simulink model "Simulatore_COMAU_UBHAND_IV_manipolazione_interna"  (Simulink 7.6)
settings: Solver=ode45  StartTime=0.0  StopTime=inf  RelTol=1e-3
values from neighbouring files (symbols this model uses):
  a_f = zeros(4,5)    [KinematicData.m]
  alpha_f = zeros(4,5)    [KinematicData.m]
  d_f = zeros(4,5)    [KinematicData.m]
  dc4_1 = 660    [KinematicData.m]
  T6HAND = [1 0 0 0;0 1 0 0; 0 0 1 357.48+95; 0 0 0 1]    [KinematicData.m]
  teta_d = atan(647.07/130)    [KinematicData.m]
  teta_f = zeros(4,5)    [KinematicData.m]
undefined symbols (not defined in the file or in its folder): a_c, a_w, alpha_c, alpha_w, d_c, d_w, Dt_fingers, teta_c
libraries: simulink x281, dspmtrx3 x171, aerolibutil x163, aerolibconvert2 x64, simulink_extras x46, vrlib x26, aerolibtransform2 x7, dspnetwork x1
(part 8 of 15)

== system Simulatore_COMAU_UBHAND_IV_manipolazione_interna/SCOPES/Direct_kinematic2/Direct_kinematic_wrist/T_0_8/Subsystem ==
  q : Inport
  alpha : Inport  Port=2
  a : Inport  Port=3
  A_01 : aerolibutil/Create 3x3 Matrix
  Constant : Constant  Value=0
  Constant1 : Constant  Value=[0 0 0 1]  VectorParams1D=off
  Constant5 : Constant  Value=d_w(1)
  Display : Display
  Gain : Gain  Gain=-1
  Gain1 : Gain  Gain=-1
  Matrix Concatenation : Concatenate  Mode=Multidimensional array  ConcatenateDimension=2
  Matrix Concatenation1 : Concatenate  Mode=Multidimensional array
  Product{,1..5} (6 alike) : Product  RndMeth=Zero
  Trigonometric Function{,1,6..8,11..12} (7 alike) : Trigonometry  Operator=cos
  Trigonometric Function{2..5,9..10,13} (7 alike) : Trigonometry
  Vector Concatenate : Concatenate  NumInputs=3  Mode=Multidimensional array
  Out1 : Outport
  wires:
  Matrix Concatenation1/1 -> Display/1, Out1/1
  Constant1/1 -> Matrix Concatenation1/2
  Matrix Concatenation/1 -> Matrix Concatenation1/1
  Vector Concatenate/1 -> Matrix Concatenation/2
  A_01/1 -> Matrix Concatenation/1
  Constant5/1 -> Vector Concatenate/3
  Product5/1 -> Vector Concatenate/2
  Product4/1 -> Vector Concatenate/1
  Trigonometric Function13/1 -> Product5/2
  Trigonometric Function12/1 -> Product4/2
  a/1 -> Product5/1, Product4/1
  Trigonometric Function11/1 -> A_01/9
  Trigonometric Function10/1 -> A_01/8
  Constant/1 -> A_01/7
  Gain1/1 -> A_01/6
  Product2/1 -> A_01/5
  Trigonometric Function5/1 -> A_01/4
  Product1/1 -> A_01/3
  Gain/1 -> A_01/2
  Trigonometric Function/1 -> A_01/1
  Product/1 -> Gain/1
  Product3/1 -> Gain1/1
  Trigonometric Function9/1 -> Product3/2
  Trigonometric Function8/1 -> Product3/1
  Trigonometric Function7/1 -> Product2/2
  Trigonometric Function6/1 -> Product2/1
  Trigonometric Function4/1 -> Product1/2
  Trigonometric Function3/1 -> Product1/1
  alpha/1 -> Trigonometric Function11/1, Trigonometric Function10/1, Trigonometric Function9/1, Trigonometric Function7/1, Trigonometric Function4/1, Trigonometric Function1/1
  q/1 -> Trigonometric Function13/1, Trigonometric Function12/1, Trigonometric Function8/1, Trigonometric Function6/1, Trigonometric Function5/1, Trigonometric Function3/1, Trigonometric Function2/1, Trigonometric Function/1
  Trigonometric Function1/1 -> Product/2
  Trigonometric Function2/1 -> Product/1

== system Simulatore_COMAU_UBHAND_IV_manipolazione_interna/SCOPES/Direct_kinematic2/Direct_kinematic_wrist/T_0_8/Subsystem1 ==
  q : Inport
  alpha : Inport  Port=2
  a : Inport  Port=3
  A_01 : aerolibutil/Create 3x3 Matrix
  Constant : Constant  Value=0
  Constant1 : Constant  Value=[0 0 0 1]  VectorParams1D=off
  Constant5 : Constant  Value=d_w(2)
  Display : Display
  Gain : Gain  Gain=-1
  Gain1 : Gain  Gain=-1
  Matrix Concatenation : Concatenate  Mode=Multidimensional array  ConcatenateDimension=2
  Matrix Concatenation1 : Concatenate  Mode=Multidimensional array
  Product{,1..5} (6 alike) : Product  RndMeth=Zero
  Trigonometric Function{,1,6..8,11..12} (7 alike) : Trigonometry  Operator=cos
  Trigonometric Function{2..5,9..10,13} (7 alike) : Trigonometry
  Vector Concatenate : Concatenate  NumInputs=3  Mode=Multidimensional array
  Out1 : Outport
  wires:
  Trigonometric Function2/1 -> Product/1
  Trigonometric Function1/1 -> Product/2
  q/1 -> Trigonometric Function/1, Trigonometric Function2/1, Trigonometric Function3/1, Trigonometric Function5/1, Trigonometric Function6/1, Trigonometric Function8/1, Trigonometric Function12/1, Trigonometric Function13/1
  alpha/1 -> Trigonometric Function1/1, Trigonometric Function4/1, Trigonometric Function7/1, Trigonometric Function9/1, Trigonometric Function10/1, Trigonometric Function11/1
  Trigonometric Function3/1 -> Product1/1
  Trigonometric Function4/1 -> Product1/2
  Trigonometric Function6/1 -> Product2/1
  Trigonometric Function7/1 -> Product2/2
  Trigonometric Function8/1 -> Product3/1
  Trigonometric Function9/1 -> Product3/2
  Product3/1 -> Gain1/1
  Product/1 -> Gain/1
  Trigonometric Function/1 -> A_01/1
  Gain/1 -> A_01/2
  Product1/1 -> A_01/3
  Trigonometric Function5/1 -> A_01/4
  Product2/1 -> A_01/5
  Gain1/1 -> A_01/6
  Constant/1 -> A_01/7
  Trigonometric Function10/1 -> A_01/8
  Trigonometric Function11/1 -> A_01/9
  a/1 -> Product5/1, Product4/1
  Trigonometric Function12/1 -> Product4/2
  Trigonometric Function13/1 -> Product5/2
  Product4/1 -> Vector Concatenate/1
  Product5/1 -> Vector Concatenate/2
  Constant5/1 -> Vector Concatenate/3
  A_01/1 -> Matrix Concatenation/1
  Vector Concatenate/1 -> Matrix Concatenation/2
  Matrix Concatenation/1 -> Matrix Concatenation1/1
  Constant1/1 -> Matrix Concatenation1/2
  Matrix Concatenation1/1 -> Out1/1, Display/1

== system Simulatore_COMAU_UBHAND_IV_manipolazione_interna/SCOPES/Direct_kinematic2/Direct_kinematic_wrist/T_0_8/wrist_reference_ ==
  Angle Conversion : aerolibconvert2/Angle Conversion  IU=deg  OU=rad
  Constant1 : Constant  Value=[0 90 0]  VectorParams1D=off
  Constant2 : Constant  Value=[0 0 0 ]'  VectorParams1D=off
  Constant4 : Constant  Value=[0 0 0 1]  VectorParams1D=off
  Matrix Concatenate : Concatenate  Mode=Multidimensional array  ConcatenateDimension=2
  Matrix Concatenate1 : Concatenate  Mode=Multidimensional array
  Rotation Angles to Direction Cosine Matrix : aerolibtransform2/Rotation Angles to Direction Cosine Matrix  rotationOrder=ZYX
  T0_W : Outport
  wires:
  Matrix Concatenate1/1 -> T0_W/1
  Rotation Angles to Direction Cosine Matrix/1 -> Matrix Concatenate/1
  Constant4/1 -> Matrix Concatenate1/2
  Matrix Concatenate/1 -> Matrix Concatenate1/1
  Angle Conversion/1 -> Rotation Angles to Direction Cosine Matrix/1
  Constant1/1 -> Angle Conversion/1
  Constant2/1 -> Matrix Concatenate/2

== system Simulatore_COMAU_UBHAND_IV_manipolazione_interna/Work Space Control/Grasp Planner/SCOPES/Direct_kinematic2/Direct_kinematic_Comau ==
  q[1..6] : Inport
  Demux3 : Demux  Outputs=6
  Matrix Multiply : Product  Multiplication=Matrix(*)
  Mux : Mux  Inputs=3
  Mux2 : Mux  Inputs=3
  T_0_3 : Subsystem
  T_3_6 : Subsystem
  T_0_6 : Outport
  wires:
  Demux3/1 -> Mux/1
  Demux3/2 -> Mux/2
  Demux3/3 -> Mux/3
  Mux/1 -> T_0_3/1(q[1..3])
  Demux3/4 -> Mux2/1
  Demux3/5 -> Mux2/2
  Demux3/6 -> Mux2/3
  Mux2/1 -> T_3_6/1(q[1..3])
  T_3_6/1(T_3_6) -> Matrix Multiply/2
  q[1..6]/1 -> Demux3/1
  T_0_3/1(T_0_3) -> Matrix Multiply/1
  Matrix Multiply/1 -> T_0_6/1

== system Simulatore_COMAU_UBHAND_IV_manipolazione_interna/Work Space Control/Grasp Planner/SCOPES/Direct_kinematic2/Direct_kinematic_hand ==
  Joint_references : Inport
  T_08 : Inport  Port=2
  Constant : Constant  Value=Dt_fingers(1,1).T_w2_finger_base  VectorParams1D=off
  Constant1 : Constant  Value=Dt_fingers(1,2).T_w2_finger_base  VectorParams1D=off
  Constant2 : Constant  Value=Dt_fingers(1,3).T_w2_finger_base  VectorParams1D=off
  Constant3 : Constant  Value=Dt_fingers(1,4).T_w2_finger_base  VectorParams1D=off
  Constant4 : Constant  Value=Dt_fingers(1,5).T_w2_finger_base  VectorParams1D=off
  Demux : Demux  Outputs=5
  Direct_kinematic_index : Subsystem
  Direct_kinematic_little : Subsystem
  Direct_kinematic_middle : Subsystem
  Direct_kinematic_ring : Subsystem
  Direct_kinematic_thumb : Subsystem
  Matrix Multiply{,1..4} (5 alike) : Product  Multiplication=Matrix(*)
  xyz{,1..4} (5 alike) : dspmtrx3/Submatrix  RowSpan=Range of rows  RowStartMode=Index  RowStartIndex=1  RowEndMode=Index  RowEndIndex=4  ColSpan=One column  ColStartMode=Index  ColStartIndex=4  ColEndMode=Index  ColEndIndex=3
  T_finger_tip_thumb : Outport
  T_finger_tip_index : Outport  Port=2
  T_finger_tip_middle : Outport  Port=3
  T_finger_tip_ring : Outport  Port=4
  T_finger_tip_little : Outport  Port=5
  T_thumb_base : Outport  Port=6
  wires:
  T_08/1 -> Matrix Multiply/1, Matrix Multiply1/1, Matrix Multiply2/1, Matrix Multiply3/1, Matrix Multiply4/1
  Constant/1 -> Matrix Multiply/2
  Constant1/1 -> Matrix Multiply1/2
  Constant2/1 -> Matrix Multiply2/2
  Constant3/1 -> Matrix Multiply3/2
  Constant4/1 -> Matrix Multiply4/2
  Matrix Multiply/1 -> Direct_kinematic_thumb/2(T_0f)
  Matrix Multiply1/1 -> Direct_kinematic_index/2(T_0f)
  Matrix Multiply2/1 -> Direct_kinematic_middle/2(T_0f)
  Matrix Multiply3/1 -> Direct_kinematic_ring/2(T_0f)
  Matrix Multiply4/1 -> Direct_kinematic_little/2(T_0f)
  Joint_references/1 -> Demux/1
  Demux/1 -> Direct_kinematic_thumb/1(Joints_reference)
  Demux/2 -> Direct_kinematic_index/1(Joints_reference)
  Demux/3 -> Direct_kinematic_middle/1(Joints_reference)
  Demux/4 -> Direct_kinematic_ring/1(Joints_reference)
  Demux/5 -> Direct_kinematic_little/1(Joints_reference)
  Direct_kinematic_index/1(T_finger_tip) -> xyz1/1
  Direct_kinematic_middle/1(T_finger_tip) -> xyz2/1
  Direct_kinematic_ring/1(T_finger_tip) -> xyz3/1
  Direct_kinematic_little/1(T_finger_tip) -> xyz4/1
  Direct_kinematic_thumb/1(T_finger_tip) -> xyz/1
  Direct_kinematic_thumb/2(T_thumb_base) -> T_thumb_base/1
  xyz1/1 -> T_finger_tip_index/1
  xyz/1 -> T_finger_tip_thumb/1
  xyz2/1 -> T_finger_tip_middle/1
  xyz3/1 -> T_finger_tip_ring/1
  xyz4/1 -> T_finger_tip_little/1

== system Simulatore_COMAU_UBHAND_IV_manipolazione_interna/Work Space Control/Grasp Planner/SCOPES/Direct_kinematic2/Direct_kinematic_wrist ==
  wrist_angles[1..2] : Inport
  T_0HAND : Inport  Port=2
  Demux3 : Demux  Outputs=2
  Mux : Mux  Inputs=2
  T_0_8 : Subsystem
  T_08 : Outport
  wires:
  T_0_8/1(T_08) -> T_08/1
  wrist_angles[1..2]/1 -> Demux3/1
  Mux/1 -> T_0_8/1(wrist_angles[1..2])
  Demux3/2 -> Mux/2
  Demux3/1 -> Mux/1
  T_0HAND/1 -> T_0_8/2(T_0HAND)

== system Simulatore_COMAU_UBHAND_IV_manipolazione_interna/Work Space Control/Grasp Planner/box/presa da destra/wrist index angles des2 ==
  Constant : Constant
  Index_medial_des : Constant  Value=80
  Index_proximal_back_forward_des : Constant  Value=50
  Index_proximal_left_right_des : Constant  Value=0
  Mux1 : Mux  Inputs=2
  Mux2 : Mux  Inputs=3
  wrist_back_forward_des : simulink/Math Operations/Slider Gain  low=-180  gain=0  high=180
  wrist_left_right_des : simulink/Math Operations/Slider Gain  low=-180  gain=0  high=180
  wrist_des : Outport
  index_des : Outport  Port=2
  wires:
  [one] -> wrist_left_right_des/1
  wrist_left_right_des/1 -> Mux1/1
  [one] -> wrist_back_forward_des/1
  wrist_back_forward_des/1 -> Mux1/2
  Index_proximal_left_right_des/1 -> Mux2/1
  Index_medial_des/1 -> Mux2/3
  Index_proximal_back_forward_des/1 -> Mux2/2
  Mux2/1 -> index_des/1
  Mux1/1 -> wrist_des/1
  Constant/1 -> [one]

== system Simulatore_COMAU_UBHAND_IV_manipolazione_interna/Work Space Control/Grasp Planner/box/presa dal basso/wrist index angles des2 ==
  Constant : Constant
  Index_medial_des : Constant  Value=70
  Index_proximal_back_forward_des : Constant  Value=70
  Index_proximal_left_right_des : Constant  Value=0
  Mux1 : Mux  Inputs=2
  Mux2 : Mux  Inputs=3
  wrist_back_forward_des : simulink/Math Operations/Slider Gain  low=-180  gain=0  high=180
  wrist_left_right_des : simulink/Math Operations/Slider Gain  low=-180  gain=0  high=180
  wrist_des : Outport
  index_des : Outport  Port=2
  wires:
  [one] -> wrist_left_right_des/1
  wrist_left_right_des/1 -> Mux1/1
  [one] -> wrist_back_forward_des/1
  wrist_back_forward_des/1 -> Mux1/2
  Index_proximal_left_right_des/1 -> Mux2/1
  Index_medial_des/1 -> Mux2/3
  Index_proximal_back_forward_des/1 -> Mux2/2
  Mux2/1 -> index_des/1
  Mux1/1 -> wrist_des/1
  Constant/1 -> [one]

== system Simulatore_COMAU_UBHAND_IV_manipolazione_interna/Work Space Control/Grasp Planner/box/presa dall'alto/wrist index angles des2 ==
  Constant : Constant
  Index_medial_des : Constant  Value=20
  Index_proximal_back_forward_des : Constant  Value=40
  Index_proximal_left_right_des : Constant  Value=5
  Mux1 : Mux  Inputs=2
  Mux2 : Mux  Inputs=3
  wrist_back_forward_des : simulink/Math Operations/Slider Gain  low=-180  gain=15  high=180
  wrist_left_right_des : simulink/Math Operations/Slider Gain  low=-180  gain=0  high=180
  wrist_des : Outport
  index_des : Outport  Port=2
  wires:
  [one] -> wrist_left_right_des/1
  wrist_left_right_des/1 -> Mux1/1
  [one] -> wrist_back_forward_des/1
  wrist_back_forward_des/1 -> Mux1/2
  Index_proximal_left_right_des/1 -> Mux2/1
  Index_medial_des/1 -> Mux2/3
  Index_proximal_back_forward_des/1 -> Mux2/2
  Mux2/1 -> index_des/1
  Mux1/1 -> wrist_des/1
  Constant/1 -> [one]

== system Simulatore_COMAU_UBHAND_IV_manipolazione_interna/Work Space Control/Grasp Planner/box/punti di contatto box/object rotation matrix ==
  box_pos : Inport
  object_hand_relative_orientation : Inport  Port=2
  object_motion_parameters : Inport  Port=3
  Constant : Constant
  Constant1 : Constant  Value=[0 0 0 1]  VectorParams1D=off
  Constant2 : Constant  Value=[0 0 0 1]  VectorParams1D=off
  Demux : Demux  Outputs=12
  Demux1 : Demux  Outputs=3
  Display{,1..3} (4 alike) : Display
  Gain : Gain  Gain=-1
  Matrix Concatenate : Concatenate  Mode=Multidimensional array  ConcatenateDimension=2
  Matrix Concatenate1 : Concatenate  Mode=Multidimensional array
  Matrix Concatenate2 : Concatenate  Mode=Multidimensional array  ConcatenateDimension=2
  Matrix Concatenate3 : Concatenate  Mode=Multidimensional array
  Matrix Multiply3 : Product  Multiplication=Matrix(*)
  Multiport Switch1 : MultiPortSwitch  Inputs=2  zeroidx=off
  Mux : Mux  Inputs=3
  Mux1 : Mux  Inputs=3
  R : Subsystem
  R_01 : Subsystem
  R_56 : Subsystem
  Ramp : simulink/Sources/Ramp  slope=50  start=0  X0=0
  definizione_T_0_object_tip1 : Subsystem
  T_object_center : Outport
  tag scope: R_0_end_effector_box=global, R_box=global, T_0_object_tip_box=global, T_rot_center_box=global, box_pos=global
  wires:
  Constant/1 -> [one]
  Constant1/1 -> Matrix Concatenate1/2
  Matrix Concatenate/1 -> Matrix Concatenate1/1
  box_pos/1 -> definizione_T_0_object_tip1/1(box_pos)
  Demux1/1 -> R_56/1(alpha)
  Demux1/2 -> R_56/2(beta)
  Demux1/3 -> R_56/3(gamma)
  object_hand_relative_orientation/1 -> Demux1/1
  object_motion_parameters/1 -> Demux/1
  Demux/1 -> R_01/1(alpha)
  Demux/2 -> R_01/2(beta)
  Demux/3 -> R_01/3(gamma)
  Demux/4 -> Mux/1
  Demux/5 -> Mux/2
  Demux/6 -> Mux/3
  Demux/7 -> Mux1/1
  Demux/8 -> Mux1/2
  Demux/9 -> Mux1/3
  R_01/1(R_01) -> definizione_T_0_object_tip1/2(R_01), [R_01]
  Mux/1 -> definizione_T_0_object_tip1/3(1pc)
  Mux1/1 -> definizione_T_0_object_tip1/4(2pr), Gain/1
  Gain/1 -> definizione_T_0_object_tip1/5(3pc)
  R_56/1(R56) -> Matrix Multiply3/2
  Matrix Multiply3/1 -> [R_0_end_effector_box]
  definizione_T_0_object_tip1/1(0pc) -> Display3/1
  definizione_T_0_object_tip1/3(0pc') -> [box_pos], Matrix Concatenate2/2
  Matrix Concatenate1/1 -> [T_rot_center_box], Display/1
  definizione_T_0_object_tip1/2(0pr) -> Matrix Concatenate/2, Display1/1
  [orientation_mode_box] -> Multiport Switch1/1
  Multiport Switch1/1 -> [R_box], Matrix Concatenate2/1
  [R_01] -> Multiport Switch1/2
  [R_05] -> Multiport Switch1/3
  Constant2/1 -> Matrix Concatenate3/2
  Matrix Concatenate2/1 -> Matrix Concatenate3/1
  Matrix Concatenate3/1 -> [T_0_object_tip_box], T_object_center/1, Display2/1
  [R_box] -> Matrix Multiply3/1
  R/1(R) -> definizione_T_0_object_tip1/6(R)
  definizione_T_0_object_tip1/5(R_05) -> [R_05]
  Demux/10 -> R/1(alpha)
  Demux/11 -> R/2(beta)
  Demux/12 -> R/3(gamma)
  definizione_T_0_object_tip1/4(R1) -> Matrix Concatenate/1

== system Simulatore_COMAU_UBHAND_IV_manipolazione_interna/Work Space Control/Grasp Planner/box/punti di contatto box/punti di contatto sfera ==
  teta/z thumb : Inport
  teta/z index : Inport  Port=2
  teta/z middle : Inport  Port=3
  teta/z ring : Inport  Port=4
  teta/z little : Inport  Port=5
  box_size : Inport  Port=6
  T_object : Inport  Port=7
  Mux : Mux  Inputs=5
  Subsystem : Subsystem
  Subsystem1 : Subsystem
  Subsystem2 : Subsystem
  Subsystem3 : Subsystem
  Subsystem4 : Subsystem
  contact_tips_xyz : Outport
  tag scope: box_size=global
  wires:
  teta/z thumb/1 -> Subsystem/1(dimens1/2/side)
  box_size/1 -> [box_size]
  teta/z middle/1 -> Subsystem2/1(dimens1/2/side)
  teta/z ring/1 -> Subsystem3/1(dimens1/2/side)
  teta/z little/1 -> Subsystem4/1(dimens1/2/side)
  Subsystem/1(xyz 0) -> Mux/1
  Subsystem1/1(xyz 0) -> Mux/2
  Subsystem2/1(xyz 0) -> Mux/3
  Subsystem3/1(xyz 0) -> Mux/4
  Subsystem4/1(xyz 0) -> Mux/5
  Mux/1 -> contact_tips_xyz/1
  T_object/1 -> Subsystem3/2(T_object), Subsystem4/2(T_object), Subsystem2/2(T_object), Subsystem1/2(T_object), Subsystem/2(T_object)
  teta/z index/1 -> Subsystem1/1(dimens1/2/side)

== system Simulatore_COMAU_UBHAND_IV_manipolazione_interna/Work Space Control/Grasp Planner/cilindro/Subsystem/R_end_effector ==
  alpha,beta,gamma : Inport
  Demux : Demux  Outputs=3
  Matrix Multiply3 : Product  Multiplication=Matrix(*)
  Matrix Multiply4 : Product  Multiplication=Matrix(*)
  rot_x : Subsystem
  rot_y : Subsystem
  rot_z : Subsystem
  R_end_effector : Outport
  wires:
  Matrix Multiply4/1 -> R_end_effector/1
  Matrix Multiply3/1 -> Matrix Multiply4/1
  rot_z/1(rotz) -> Matrix Multiply4/2
  rot_y/1(roty) -> Matrix Multiply3/2
  rot_x/1(rotx) -> Matrix Multiply3/1
  Demux/1 -> rot_x/1(alpha)
  Demux/2 -> rot_y/1(beta)
  Demux/3 -> rot_z/1(gamma)
  alpha,beta,gamma/1 -> Demux/1

== system Simulatore_COMAU_UBHAND_IV_manipolazione_interna/Work Space Control/Grasp Planner/cilindro/presa da destra/wrist index angles des2 ==
  Constant : Constant
  Index_medial_des : Constant  Value=50
  Index_proximal_back_forward_des : Constant  Value=30
  Index_proximal_left_right_des : Constant  Value=10
  Mux1 : Mux  Inputs=2
  Mux2 : Mux  Inputs=3
  wrist_back_forward_des : simulink/Math Operations/Slider Gain  low=-180  gain=0  high=180
  wrist_left_right_des : simulink/Math Operations/Slider Gain  low=-180  gain=0  high=180
  wrist_des : Outport
  index_des : Outport  Port=2
  wires:
  [one] -> wrist_left_right_des/1
  wrist_left_right_des/1 -> Mux1/1
  [one] -> wrist_back_forward_des/1
  wrist_back_forward_des/1 -> Mux1/2
  Index_proximal_left_right_des/1 -> Mux2/1
  Index_medial_des/1 -> Mux2/3
  Index_proximal_back_forward_des/1 -> Mux2/2
  Mux2/1 -> index_des/1
  Mux1/1 -> wrist_des/1
  Constant/1 -> [one]

== system Simulatore_COMAU_UBHAND_IV_manipolazione_interna/Work Space Control/Grasp Planner/cilindro/presa dal basso/wrist index angles des2 ==
  Constant : Constant
  Index_medial_des : Constant  Value=15
  Index_proximal_back_forward_des : Constant  Value=80
  Index_proximal_left_right_des : Constant  Value=0
  Mux1 : Mux  Inputs=2
  Mux2 : Mux  Inputs=3
  wrist_back_forward_des : simulink/Math Operations/Slider Gain  low=-180  gain=0  high=180
  wrist_left_right_des : simulink/Math Operations/Slider Gain  low=-180  gain=0  high=180
  wrist_des : Outport
  index_des : Outport  Port=2
  wires:
  [one] -> wrist_left_right_des/1
  wrist_left_right_des/1 -> Mux1/1
  [one] -> wrist_back_forward_des/1
  wrist_back_forward_des/1 -> Mux1/2
  Index_proximal_left_right_des/1 -> Mux2/1
  Index_medial_des/1 -> Mux2/3
  Index_proximal_back_forward_des/1 -> Mux2/2
  Mux2/1 -> index_des/1
  Mux1/1 -> wrist_des/1
  Constant/1 -> [one]

== system Simulatore_COMAU_UBHAND_IV_manipolazione_interna/Work Space Control/Grasp Planner/cilindro/presa dall'alto/wrist index angles des2 ==
  Constant : Constant
  Index_medial_des : Constant  Value=50
  Index_proximal_back_forward_des : Constant  Value=20
  Index_proximal_left_right_des : Constant  Value=10
  Mux1 : Mux  Inputs=2
  Mux2 : Mux  Inputs=3
  wrist_back_forward_des : simulink/Math Operations/Slider Gain  low=-180  gain=28.8  high=180
  wrist_left_right_des : simulink/Math Operations/Slider Gain  low=-180  gain=0  high=180
  wrist_des : Outport
  index_des : Outport  Port=2
  wires:
  [one] -> wrist_left_right_des/1
  wrist_left_right_des/1 -> Mux1/1
  [one] -> wrist_back_forward_des/1
  wrist_back_forward_des/1 -> Mux1/2
  Index_proximal_left_right_des/1 -> Mux2/1
  Index_medial_des/1 -> Mux2/3
  Index_proximal_back_forward_des/1 -> Mux2/2
  Mux2/1 -> index_des/1
  Mux1/1 -> wrist_des/1
  Constant/1 -> [one]

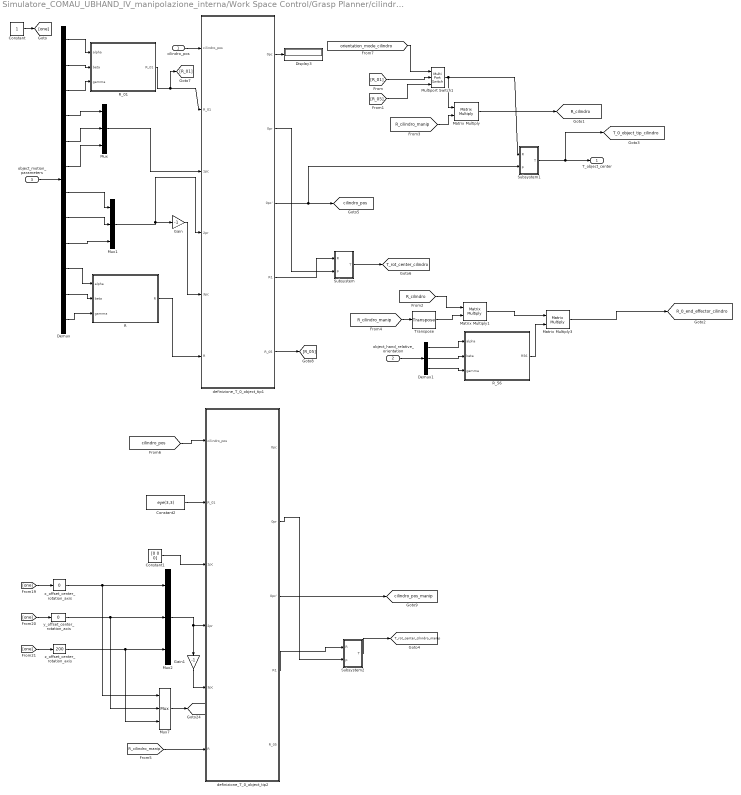
== system Simulatore_COMAU_UBHAND_IV_manipolazione_interna/Work Space Control/Grasp Planner/cilindro/punti di contatto cilindro/object rotation matrix ==
  cilindro_pos : Inport
  object_hand_relative_orientation : Inport  Port=2
  object_motion_parameters : Inport  Port=3
  Constant : Constant
  Constant1 : Constant  Value=[0 0 0]
  Constant2 : Constant  Value=eye(3,3)
  Demux : Demux  Outputs=12
  Demux1 : Demux  Outputs=3
  Display3 : Display
  Gain : Gain  Gain=-1
  Gain1 : Gain  Gain=-1
  Matrix Multiply : Product  Multiplication=Matrix(*)
  Matrix Multiply1 : Product  Multiplication=Matrix(*)
  Matrix Multiply3 : Product  Multiplication=Matrix(*)
  Multiport Switch1 : MultiPortSwitch  Inputs=2  zeroidx=off
  Mux{,1..2,7} (4 alike) : Mux  Inputs=3
  R : Subsystem
  R_01 : Subsystem
  R_56 : Subsystem
  Subsystem : Subsystem
  Subsystem1 : Subsystem
  Subsystem2 : Subsystem
  Transpose : dspmtrx3/Transpose  Hermitian=off  overflowFlag=off
  definizione_T_0_object_tip1 : Subsystem
  definizione_T_0_object_tip2 : Subsystem
  x_offset_center_rotation_axis : simulink/Math Operations/Slider Gain  low=0  gain=0  high=500
  y_offset_center_rotation_axis : simulink/Math Operations/Slider Gain  low=0  gain=0  high=500
  z_offset_center_rotation_axis : simulink/Math Operations/Slider Gain  low=-1000  gain=200  high=500
  T_object_center : Outport
  tag scope: R_0_end_effector_cilindro=global, R_cilindro=global, T_0_object_tip_cilindro=global, T_rot_center_cilindro=global, T_rot_center_cilindro_manip=global, cilindro_pos=global, cilindro_pos_manip=global, reference_point=global
  wires:
  Constant/1 -> [one]
  cilindro_pos/1 -> definizione_T_0_object_tip1/1(cilindro_pos)
  Demux1/1 -> R_56/1(alpha)
  Demux1/2 -> R_56/2(beta)
  Demux1/3 -> R_56/3(gamma)
  object_hand_relative_orientation/1 -> Demux1/1
  object_motion_parameters/1 -> Demux/1
  Demux/4 -> Mux/1
  Demux/5 -> Mux/2
  Demux/6 -> Mux/3
  Demux/7 -> Mux1/1
  Demux/8 -> Mux1/2
  Demux/9 -> Mux1/3
  R_01/1(R_01) -> definizione_T_0_object_tip1/2(R_01), [R_01]
  Mux/1 -> definizione_T_0_object_tip1/3(1pc)
  Mux1/1 -> definizione_T_0_object_tip1/4(2pr), Gain/1
  Gain/1 -> definizione_T_0_object_tip1/5(3pc)
  R_56/1(R56) -> Matrix Multiply3/2
  Matrix Multiply3/1 -> [R_0_end_effector_cilindro]
  definizione_T_0_object_tip1/1(0pc) -> Display3/1
  definizione_T_0_object_tip1/3(0pc') -> Subsystem1/2(p), [cilindro_pos]
  Subsystem/1(T) -> [T_rot_center_cilindro]
  definizione_T_0_object_tip1/2(0pr) -> Subsystem/2(p)
  [orientation_mode_cilindro] -> Multiport Switch1/1
  [R_01] -> Multiport Switch1/2
  [R_05] -> Multiport Switch1/3
  Subsystem1/1(T) -> [T_0_object_tip_cilindro], T_object_center/1
  R/1(R) -> definizione_T_0_object_tip1/6(R)
  definizione_T_0_object_tip1/5(R_05) -> [R_05]
  Demux/10 -> R/1(alpha)
  Demux/11 -> R/2(beta)
  Demux/12 -> R/3(gamma)
  definizione_T_0_object_tip1/4(R1) -> Subsystem/1(R)
  [R_cilindro_manip] -> Matrix Multiply/2
  Multiport Switch1/1 -> Matrix Multiply/1, Subsystem1/1(R)
  Matrix Multiply/1 -> [R_cilindro]
  [R_cilindro_manip] -> Transpose/1
  Matrix Multiply1/1 -> Matrix Multiply3/1
  [R_cilindro] -> Matrix Multiply1/1
  Transpose/1 -> Matrix Multiply1/2
  Demux/1 -> R_01/1(alpha)
  Demux/2 -> R_01/2(beta)
  Demux/3 -> R_01/3(gamma)
  [one] -> x_offset_center_rotation_axis/1
  [one] -> y_offset_center_rotation_axis/1
  [one] -> z_offset_center_rotation_axis/1
  x_offset_center_rotation_axis/1 -> Mux2/1, Mux7/1
  y_offset_center_rotation_axis/1 -> Mux2/2, Mux7/2
  z_offset_center_rotation_axis/1 -> Mux2/3, Mux7/3
  Mux2/1 -> definizione_T_0_object_tip2/4(2pr), Gain1/1
  Gain1/1 -> definizione_T_0_object_tip2/5(3pc)
  [R_cilindro_manip] -> definizione_T_0_object_tip2/6(R)
  Constant2/1 -> definizione_T_0_object_tip2/2(R_01)
  definizione_T_0_object_tip2/3(0pc') -> [cilindro_pos_manip]
  Mux7/1 -> [reference_point]
  Constant1/1 -> definizione_T_0_object_tip2/3(1pc)
  [cilindro_pos] -> definizione_T_0_object_tip2/1(cilindro_pos)
  Subsystem2/1(T) -> [T_rot_center_cilindro_manip]
  definizione_T_0_object_tip2/2(0pr) -> Subsystem2/2(p)
  definizione_T_0_object_tip2/4(R1) -> Subsystem2/1(R)

== system Simulatore_COMAU_UBHAND_IV_manipolazione_interna/Work Space Control/Grasp Planner/cilindro/punti di contatto cilindro/punti di contatto cilindro ==
  teta/z thumb : Inport
  teta/z index : Inport  Port=2
  teta/z middle : Inport  Port=3
  teta/z ring : Inport  Port=4
  teta/z little : Inport  Port=5
  cilindro_size : Inport  Port=6
  T_object : Inport  Port=7
  offset : Inport  Port=8
  object_manipulation : Inport  Port=9
  Display : Display
  Display1 : Display
  Mux : Mux  Inputs=5
  Subsystem1 : Subsystem
  Subsystem2 : Subsystem
  Subsystem3 : Subsystem
  Subsystem4 : Subsystem
  Subsystem5 : Subsystem
  contact_tips_xyz : Outport
  index_xyz_0 : Outport  Port=2
  tag scope: cilindro_size=global
  wires:
  teta/z thumb/1 -> Subsystem2/1(teta/z)
  cilindro_size/1 -> [cilindro_size]
  teta/z index/1 -> Subsystem1/1(teta/z)
  Mux/1 -> contact_tips_xyz/1, Display/1
  teta/z middle/1 -> Subsystem3/1(teta/z)
  teta/z ring/1 -> Subsystem4/1(teta/z)
  teta/z little/1 -> Subsystem5/1(teta/z)
  T_object/1 -> Subsystem1/2(T_object), Subsystem2/2(T_object), Subsystem3/2(T_object), Subsystem4/2(T_object), Subsystem5/2(T_object)
  offset/1 -> Subsystem2/3(offset), Subsystem1/3(offset), Subsystem3/3(offset), Subsystem4/3(offset), Subsystem5/3(offset)
  Subsystem2/1(xyz 0) -> Mux/1
  Subsystem5/1(xyz 0) -> Mux/5
  Subsystem4/1(xyz 0) -> Mux/4
  Subsystem3/1(xyz 0) -> Mux/3
  Subsystem1/2(xyz 0) -> Mux/2
  Subsystem1/1(index_xyz_0) -> index_xyz_0/1, Display1/1
  object_manipulation/1 -> Subsystem2/4(object_manipulation), Subsystem4/4(object_manipulation), Subsystem5/4(object_manipulation), Subsystem3/4(object_manipulation), Subsystem1/4(object_manipulation)

== system Simulatore_COMAU_UBHAND_IV_manipolazione_interna/Work Space Control/Grasp Planner/sfera/presa dall'alto/wrist index angles des2 ==
  Constant : Constant
  Index_medial_des : Constant  Value=30
  Index_proximal_back_forward_des : Constant  Value=0
  Index_proximal_left_right_des : Constant  Value=20
  Mux1 : Mux  Inputs=2
  Mux2 : Mux  Inputs=3
  wrist_back_forward_des : simulink/Math Operations/Slider Gain  low=-180  gain=0  high=180
  wrist_left_right_des : simulink/Math Operations/Slider Gain  low=-180  gain=0  high=180
  wrist_des : Outport
  index_des : Outport  Port=2
  wires:
  Constant/1 -> [one]
  Mux1/1 -> wrist_des/1
  Mux2/1 -> index_des/1
  Index_proximal_back_forward_des/1 -> Mux2/2
  Index_medial_des/1 -> Mux2/3
  Index_proximal_left_right_des/1 -> Mux2/1
  wrist_back_forward_des/1 -> Mux1/2
  [one] -> wrist_back_forward_des/1
  wrist_left_right_des/1 -> Mux1/1
  [one] -> wrist_left_right_des/1

== system Simulatore_COMAU_UBHAND_IV_manipolazione_interna/Work Space Control/Grasp Planner/sfera/punti di contatto sfera/object rotation matrix ==
  sfera_pos : Inport
  object_hand_relative_orientation : Inport  Port=2
  object_motion_parameters : Inport  Port=3
  Constant : Constant
  Constant1 : Constant  Value=[0 0 0 1]  VectorParams1D=off
  Constant2 : Constant  Value=[0 0 0 1]  VectorParams1D=off
  Demux : Demux  Outputs=12
  Demux1 : Demux  Outputs=3
  Display{,1..3} (4 alike) : Display
  Gain : Gain  Gain=-1
  Matrix Concatenate : Concatenate  Mode=Multidimensional array  ConcatenateDimension=2
  Matrix Concatenate1 : Concatenate  Mode=Multidimensional array
  Matrix Concatenate2 : Concatenate  Mode=Multidimensional array  ConcatenateDimension=2
  Matrix Concatenate3 : Concatenate  Mode=Multidimensional array
  Matrix Multiply3 : Product  Multiplication=Matrix(*)
  Multiport Switch1 : MultiPortSwitch  Inputs=2  zeroidx=off
  Mux : Mux  Inputs=3
  Mux1 : Mux  Inputs=3
  R : Subsystem
  R_01 : Subsystem
  R_56 : Subsystem
  Ramp : simulink/Sources/Ramp  slope=50  start=0  X0=0
  definizione_T_0_object_tip1 : Subsystem
  T_object_center : Outport
  tag scope: R_0_end_effector_sfera=global, R_sfera=global, T_0_object_tip_sfera=global, T_rot_center_sfera=global, sfera_pos=global
  wires:
  Constant/1 -> [one]
  Constant1/1 -> Matrix Concatenate1/2
  Matrix Concatenate/1 -> Matrix Concatenate1/1
  sfera_pos/1 -> definizione_T_0_object_tip1/1(sfera_pos)
  Demux1/1 -> R_56/1(alpha)
  Demux1/2 -> R_56/2(beta)
  Demux1/3 -> R_56/3(gamma)
  object_hand_relative_orientation/1 -> Demux1/1
  object_motion_parameters/1 -> Demux/1
  Demux/1 -> R_01/1(alpha)
  Demux/2 -> R_01/2(beta)
  Demux/3 -> R_01/3(gamma)
  Demux/4 -> Mux/1
  Demux/5 -> Mux/2
  Demux/6 -> Mux/3
  Demux/7 -> Mux1/1
  Demux/8 -> Mux1/2
  Demux/9 -> Mux1/3
  R_01/1(R_01) -> definizione_T_0_object_tip1/2(R_01), [R_01]
  Mux/1 -> definizione_T_0_object_tip1/3(1pc)
  Mux1/1 -> definizione_T_0_object_tip1/4(2pr), Gain/1
  Gain/1 -> definizione_T_0_object_tip1/5(3pc)
  R_56/1(R56) -> Matrix Multiply3/2
  Matrix Multiply3/1 -> [R_0_end_effector_sfera]
  definizione_T_0_object_tip1/1(0pc) -> Display3/1
  definizione_T_0_object_tip1/3(0pc') -> [sfera_pos], Matrix Concatenate2/2
  Matrix Concatenate1/1 -> [T_rot_center_sfera], Display/1
  definizione_T_0_object_tip1/2(0pr) -> Matrix Concatenate/2, Display1/1
  [orientation_mode_sfera] -> Multiport Switch1/1
  Multiport Switch1/1 -> [R_sfera], Matrix Concatenate2/1
  [R_01] -> Multiport Switch1/2
  [R_05] -> Multiport Switch1/3
  Constant2/1 -> Matrix Concatenate3/2
  Matrix Concatenate2/1 -> Matrix Concatenate3/1
  Matrix Concatenate3/1 -> [T_0_object_tip_sfera], T_object_center/1, Display2/1
  [R_sfera] -> Matrix Multiply3/1
  R/1(R) -> definizione_T_0_object_tip1/6(R)
  definizione_T_0_object_tip1/5(R_05) -> [R_05]
  Demux/10 -> R/1(alpha)
  Demux/11 -> R/2(beta)
  Demux/12 -> R/3(gamma)
  definizione_T_0_object_tip1/4(R1) -> Matrix Concatenate/1

== system Simulatore_COMAU_UBHAND_IV_manipolazione_interna/Work Space Control/Grasp Planner/sfera/punti di contatto sfera/punti di contatto sfera ==
  teta/z thumb : Inport
  teta/z index : Inport  Port=2
  teta/z middle : Inport  Port=3
  teta/z ring : Inport  Port=4
  teta/z little : Inport  Port=5
  sfera_size : Inport  Port=6
  T_object : Inport  Port=7
  Constant : Constant
  Matrix Multiply3 : Product  Multiplication=Matrix(*)
  Multiport Switch3 : MultiPortSwitch  zeroidx=off
  Mux : Mux  Inputs=5
  Mux1 : Mux  Inputs=3
  Mux2 : Mux  Inputs=3
  Mux3 : Mux  Inputs=3
  R : Subsystem
  Subsystem : Subsystem
  Subsystem1 : Subsystem
  Subsystem2 : Subsystem
  Subsystem3 : Subsystem
  Subsystem4 : Subsystem
  alpha_relative_orientation : simulink/Math Operations/Slider Gain  low=-180  gain=90  high=180
  alpha_relative_orientation1 : simulink/Math Operations/Slider Gain  low=-180  gain=180  high=180
  alpha_relative_orientation2 : simulink/Math Operations/Slider Gain  low=-180  gain=0  high=180
  beta_relative_orientation : simulink/Math Operations/Slider Gain  low=-180  gain=0  high=180
  beta_relative_orientation1 : simulink/Math Operations/Slider Gain  low=-180  gain=0  high=180
  beta_relative_orientation2 : simulink/Math Operations/Slider Gain  low=-180  gain=0  high=180
  gamma_relative_orientation : simulink/Math Operations/Slider Gain  low=-180  gain=0  high=180
  gamma_relative_orientation1 : simulink/Math Operations/Slider Gain  low=-180  gain=0  high=180
  gamma_relative_orientation2 : simulink/Math Operations/Slider Gain  low=-180  gain=0  high=180
  contact_tips_xyz : Outport
  tag scope: sfera_size=global
  wires:
  teta/z thumb/1 -> Subsystem/1(teta/phi)
  sfera_size/1 -> [sfera_size]
  teta/z index/1 -> Subsystem1/1(teta/phi)
  teta/z middle/1 -> Subsystem2/1(teta/phi)
  teta/z ring/1 -> Subsystem3/1(teta/phi)
  teta/z little/1 -> Subsystem4/1(teta/phi)
  Mux/1 -> contact_tips_xyz/1
  Subsystem/1(xyz 0) -> Mux/1
  Subsystem1/1(xyz 0) -> Mux/2
  Subsystem2/1(xyz 0) -> Mux/3
  Subsystem3/1(xyz 0) -> Mux/4
  Subsystem4/1(xyz 0) -> Mux/5
  Mux2/1 -> Multiport Switch3/3
  gamma_relative_orientation/1 -> Mux2/3
  beta_relative_orientation/1 -> Mux2/2
  alpha_relative_orientation/1 -> Mux2/1
  [one] -> gamma_relative_orientation/1
  [one] -> beta_relative_orientation/1
  [one] -> alpha_relative_orientation/1
  gamma_relative_orientation1/1 -> Mux1/3
  beta_relative_orientation1/1 -> Mux1/2
  alpha_relative_orientation1/1 -> Mux1/1
  [one] -> gamma_relative_orientation1/1
  [one] -> beta_relative_orientation1/1
  [one] -> alpha_relative_orientation1/1
  Mux1/1 -> Multiport Switch3/4
  gamma_relative_orientation2/1 -> Mux3/3
  beta_relative_orientation2/1 -> Mux3/2
  alpha_relative_orientation2/1 -> Mux3/1
  [one] -> gamma_relative_orientation2/1
  [one] -> beta_relative_orientation2/1
  [one] -> alpha_relative_orientation2/1
  Multiport Switch3/1 -> R/1(alpha,beta,gamma)
  Mux3/1 -> Multiport Switch3/2
  [tipo_di_presa] -> Multiport Switch3/1
  T_object/1 -> Matrix Multiply3/1
  R/1(T) -> Matrix Multiply3/2
  Matrix Multiply3/1 -> [T_object]
  [T_object] -> Subsystem/2(T_object), Subsystem1/2(T_object), Subsystem2/2(T_object), Subsystem3/2(T_object), Subsystem4/2(T_object)
  Constant/1 -> [one]

== system Simulatore_COMAU_UBHAND_IV_manipolazione_interna/Work Space Control/Inverse_kinematic/Inverse_kinematic_comau/R_0index/T_0index ==
  wrist_angles_des : Inport
  index_angles_des : Inport  Port=2
  T_0_end_effector : Inport  Port=3
  Constant : Constant  Value=[0 0 0 1]  VectorParams1D=off
  Constant1 : Constant  Value=Dt_fingers(2).T_w2_finger_base  VectorParams1D=off
  Direct_kinematic_index : Subsystem
  Direct_kinematic_wrist : Subsystem
  Display : Display
  Matrix Concatenate : Concatenate  Mode=Multidimensional array  ConcatenateDimension=2
  Matrix Concatenate1 : Concatenate  Mode=Multidimensional array
  Matrix Multiply1 : Product  Multiplication=Matrix(*)
  R_0EE : dspmtrx3/Submatrix  RowSpan=Range of rows  RowStartMode=Index  RowStartIndex=1  RowEndMode=Index  RowEndIndex=3  ColSpan=Range of columns  ColStartMode=Index  ColStartIndex=1  ColEndMode=Index  ColEndIndex=3
  R_0EE1 : dspmtrx3/Submatrix  RowSpan=Range of rows  RowStartMode=Index  RowStartIndex=1  RowEndMode=Index  RowEndIndex=3  ColSpan=One column  ColStartMode=Index  ColStartIndex=4  ColEndMode=Index  ColEndIndex=3
  R_0EE2 : dspmtrx3/Submatrix  RowSpan=Range of rows  RowStartMode=Index  RowStartIndex=1  RowEndMode=Index  RowEndIndex=3  ColSpan=Range of columns  ColStartMode=Index  ColStartIndex=1  ColEndMode=Index  ColEndIndex=3
  T_0index : Outport
  wires:
  Constant1/1 -> R_0EE/1
  Matrix Multiply1/1 -> Direct_kinematic_index/2(R_0f)
  Direct_kinematic_wrist/1(R_08) -> Matrix Multiply1/1
  index_angles_des/1 -> Direct_kinematic_index/1(Joints_reference)
  wrist_angles_des/1 -> Direct_kinematic_wrist/2(wrist_angles[1..2])
  R_0EE/1 -> Matrix Multiply1/2
  Constant/1 -> Matrix Concatenate1/2
  Matrix Concatenate/1 -> Matrix Concatenate1/1
  Direct_kinematic_index/1(R_0index) -> Matrix Concatenate/1
  R_0EE1/1 -> Matrix Concatenate/2
  Matrix Concatenate1/1 -> T_0index/1
  T_0_end_effector/1 -> R_0EE2/1, R_0EE1/1
  R_0EE2/1 -> Direct_kinematic_wrist/1(R_0H)

== system Simulatore_COMAU_UBHAND_IV_manipolazione_interna/Work Space Control/Inverse_kinematic/Inverse_kinematic_comau/R_0index/T_6_index_^-1 ==
  wrist_angles_des : Inport
  index_angles_des : Inport  Port=2
  Constant2 : Constant  Value=Dt_fingers(2).T_w2_finger_base  VectorParams1D=off
  Direct_kinematic_index1 : Subsystem
  Direct_kinematic_wrist1 : Subsystem
  Matrix Multiply2 : Product  Multiplication=Matrix(*)
  T6H : Constant  Value=T6HAND  VectorParams1D=off
  T^-1 : Subsystem
  T_6_index^-1 : Outport
  wires:
  Matrix Multiply2/1 -> Direct_kinematic_index1/2(T_0f)
  Direct_kinematic_wrist1/1(T_68) -> Matrix Multiply2/1
  Direct_kinematic_index1/1(T_6_index) -> T^-1/1(T)
  wrist_angles_des/1 -> Direct_kinematic_wrist1/2(wrist_angles[1..2])
  index_angles_des/1 -> Direct_kinematic_index1/1(Joints_reference)
  T6H/1 -> Direct_kinematic_wrist1/1(T_6H)
  Constant2/1 -> Matrix Multiply2/2
  T^-1/1(T^-1) -> T_6_index^-1/1

== system Simulatore_COMAU_UBHAND_IV_manipolazione_interna/Work Space Control/Inverse_kinematic/Inverse_kinematic_comau/R_0index/T_6_object^-1 ==
  wrist_angles_des : Inport
  index_angles_des : Inport  Port=2
  T_index_object : Inport  Port=3
  Constant2 : Constant  Value=Dt_fingers(2).T_w2_finger_base  VectorParams1D=off
  Direct_kinematic_index1 : Subsystem
  Direct_kinematic_wrist1 : Subsystem
  Matrix Multiply1 : Product  Multiplication=Matrix(*)
  Matrix Multiply2 : Product  Multiplication=Matrix(*)
  T6H : Constant  Value=T6HAND  VectorParams1D=off
  T^-1 : Subsystem
  T_6_object^-1 : Outport
  wires:
  T^-1/1(T^-1) -> T_6_object^-1/1
  Constant2/1 -> Matrix Multiply2/2
  T6H/1 -> Direct_kinematic_wrist1/1(T_6H)
  index_angles_des/1 -> Direct_kinematic_index1/1(Joints_reference)
  wrist_angles_des/1 -> Direct_kinematic_wrist1/2(wrist_angles[1..2])
  Direct_kinematic_wrist1/1(T_68) -> Matrix Multiply2/1
  Matrix Multiply2/1 -> Direct_kinematic_index1/2(T_0f)
  Direct_kinematic_index1/1(T_6_index) -> Matrix Multiply1/1
  T_index_object/1 -> Matrix Multiply1/2
  Matrix Multiply1/1 -> T^-1/1(T)

== system Simulatore_COMAU_UBHAND_IV_manipolazione_interna/Work Space Control/Inverse_kinematic/Inverse_kinematic_comau/R_0index/T_index_object ==
  T_0_index : Inport
  T_0_object : Inport  Port=2
  Display : Display
  Matrix Multiply1 : Product  Multiplication=Matrix(*)
  T^-1 : Subsystem
  T_index_object : Outport
  wires:
  Matrix Multiply1/1 -> T_index_object/1
  T_0_index/1 -> T^-1/1(T)
  T^-1/1(T^-1) -> Matrix Multiply1/1
  T_0_object/1 -> Matrix Multiply1/2, Display/1

== system Simulatore_COMAU_UBHAND_IV_manipolazione_interna/Work Space Control/Inverse_kinematic/Inverse_kinematic_comau/Subsystem/q5 appartiene a [-180 0] ==
  R_36 : Inport
  Mux : Mux  Inputs=3
  q4 : Subsystem
  q5 : Subsystem
  q6 : Subsystem
  q_[4..6] : Outport
  wires:
  Mux/1 -> q_[4..6]/1
  q6/1(q_6) -> Mux/3
  q5/1(q_5) -> Mux/2
  q4/1(q_4) -> Mux/1
  R_36/1 -> q6/1(R_36), q5/1(R_36), q4/1(R_36)

== system Simulatore_COMAU_UBHAND_IV_manipolazione_interna/Work Space Control/Inverse_kinematic/Inverse_kinematic_comau/Subsystem/q5 appartiene a [0 180] ==
  R_36 : Inport
  Mux : Mux  Inputs=3
  q4 : Subsystem
  q5 : Subsystem
  q6 : Subsystem
  q_[4..6] : Outport
  wires:
  R_36/1 -> q4/1(R_36), q5/1(R_36), q6/1(R_36)
  q4/1(q_4) -> Mux/1
  q5/1(q_5) -> Mux/2
  q6/1(q_6) -> Mux/3
  Mux/1 -> q_[4..6]/1

== system Simulatore_COMAU_UBHAND_IV_manipolazione_interna/Work Space Control/Inverse_kinematic/Inverse_kinematic_comau/T_0_3/Subsystem ==
  q : Inport
  alpha : Inport  Port=2
  a : Inport  Port=3
  A_01 : aerolibutil/Create 3x3 Matrix
  Constant : Constant  Value=0
  Constant1 : Constant  Value=[0 0 0 1]  VectorParams1D=off
  Constant5 : Constant  Value=d_c(1)
  Display : Display
  Gain : Gain  Gain=-1
  Gain1 : Gain  Gain=-1
  Matrix Concatenation : Concatenate  Mode=Multidimensional array  ConcatenateDimension=2
  Matrix Concatenation1 : Concatenate  Mode=Multidimensional array
  Product{,1..5} (6 alike) : Product  RndMeth=Zero
  Trigonometric Function{,1,6..8,11..12} (7 alike) : Trigonometry  Operator=cos
  Trigonometric Function{2..5,9..10,13} (7 alike) : Trigonometry
  Vector Concatenate : Concatenate  NumInputs=3  Mode=Multidimensional array
  Out1 : Outport
  wires:
  Trigonometric Function2/1 -> Product/1
  Trigonometric Function1/1 -> Product/2
  q/1 -> Trigonometric Function/1, Trigonometric Function2/1, Trigonometric Function3/1, Trigonometric Function5/1, Trigonometric Function6/1, Trigonometric Function8/1, Trigonometric Function12/1, Trigonometric Function13/1
  alpha/1 -> Trigonometric Function1/1, Trigonometric Function4/1, Trigonometric Function7/1, Trigonometric Function9/1, Trigonometric Function10/1, Trigonometric Function11/1
  Trigonometric Function3/1 -> Product1/1
  Trigonometric Function4/1 -> Product1/2
  Trigonometric Function6/1 -> Product2/1
  Trigonometric Function7/1 -> Product2/2
  Trigonometric Function8/1 -> Product3/1
  Trigonometric Function9/1 -> Product3/2
  Product3/1 -> Gain1/1
  Product/1 -> Gain/1
  Trigonometric Function/1 -> A_01/1
  Gain/1 -> A_01/2
  Product1/1 -> A_01/3
  Trigonometric Function5/1 -> A_01/4
  Product2/1 -> A_01/5
  Gain1/1 -> A_01/6
  Constant/1 -> A_01/7
  Trigonometric Function10/1 -> A_01/8
  Trigonometric Function11/1 -> A_01/9
  a/1 -> Product4/1, Product5/1
  Trigonometric Function12/1 -> Product4/2
  Trigonometric Function13/1 -> Product5/2
  Product4/1 -> Vector Concatenate/1
  Product5/1 -> Vector Concatenate/2
  Constant5/1 -> Vector Concatenate/3
  A_01/1 -> Matrix Concatenation/1
  Vector Concatenate/1 -> Matrix Concatenation/2
  Matrix Concatenation/1 -> Matrix Concatenation1/1
  Constant1/1 -> Matrix Concatenation1/2
  Matrix Concatenation1/1 -> Out1/1, Display/1

== system Simulatore_COMAU_UBHAND_IV_manipolazione_interna/Work Space Control/Inverse_kinematic/Inverse_kinematic_comau/T_0_3/Subsystem1 ==
  q : Inport
  alpha : Inport  Port=2
  a : Inport  Port=3
  A_01 : aerolibutil/Create 3x3 Matrix
  Constant : Constant  Value=0
  Constant1 : Constant  Value=[0 0 0 1]  VectorParams1D=off
  Constant5 : Constant  Value=d_c(2)
  Display : Display
  Gain : Gain  Gain=-1
  Gain1 : Gain  Gain=-1
  Matrix Concatenation : Concatenate  Mode=Multidimensional array  ConcatenateDimension=2
  Matrix Concatenation1 : Concatenate  Mode=Multidimensional array
  Product{,1..5} (6 alike) : Product  RndMeth=Zero
  Trigonometric Function{,1,6..8,11..12} (7 alike) : Trigonometry  Operator=cos
  Trigonometric Function{2..5,9..10,13} (7 alike) : Trigonometry
  Vector Concatenate : Concatenate  NumInputs=3  Mode=Multidimensional array
  Out1 : Outport
  wires:
  Matrix Concatenation1/1 -> Display/1, Out1/1
  Constant1/1 -> Matrix Concatenation1/2
  Matrix Concatenation/1 -> Matrix Concatenation1/1
  Vector Concatenate/1 -> Matrix Concatenation/2
  A_01/1 -> Matrix Concatenation/1
  Constant5/1 -> Vector Concatenate/3
  Product5/1 -> Vector Concatenate/2
  Product4/1 -> Vector Concatenate/1
  Trigonometric Function13/1 -> Product5/2
  Trigonometric Function12/1 -> Product4/2
  a/1 -> Product4/1, Product5/1
  Trigonometric Function11/1 -> A_01/9
  Trigonometric Function10/1 -> A_01/8
  Constant/1 -> A_01/7
  Gain1/1 -> A_01/6
  Product2/1 -> A_01/5
  Trigonometric Function5/1 -> A_01/4
  Product1/1 -> A_01/3
  Gain/1 -> A_01/2
  Trigonometric Function/1 -> A_01/1
  Product/1 -> Gain/1
  Product3/1 -> Gain1/1
  Trigonometric Function9/1 -> Product3/2
  Trigonometric Function8/1 -> Product3/1
  Trigonometric Function7/1 -> Product2/2
  Trigonometric Function6/1 -> Product2/1
  Trigonometric Function4/1 -> Product1/2
  Trigonometric Function3/1 -> Product1/1
  alpha/1 -> Trigonometric Function11/1, Trigonometric Function10/1, Trigonometric Function9/1, Trigonometric Function7/1, Trigonometric Function4/1, Trigonometric Function1/1
  q/1 -> Trigonometric Function13/1, Trigonometric Function12/1, Trigonometric Function8/1, Trigonometric Function6/1, Trigonometric Function5/1, Trigonometric Function3/1, Trigonometric Function2/1, Trigonometric Function/1
  Trigonometric Function1/1 -> Product/2
  Trigonometric Function2/1 -> Product/1

== system Simulatore_COMAU_UBHAND_IV_manipolazione_interna/Work Space Control/Inverse_kinematic/Inverse_kinematic_comau/T_0_3/Subsystem2 ==
  q : Inport
  alpha : Inport  Port=2
  a : Inport  Port=3
  A_01 : aerolibutil/Create 3x3 Matrix
  Constant : Constant  Value=0
  Constant1 : Constant  Value=[0 0 0 1]  VectorParams1D=off
  Constant5 : Constant  Value=d_c(3)
  Display : Display
  Gain : Gain  Gain=-1
  Gain1 : Gain  Gain=-1
  Matrix Concatenation : Concatenate  Mode=Multidimensional array  ConcatenateDimension=2
  Matrix Concatenation1 : Concatenate  Mode=Multidimensional array
  Product{,1..5} (6 alike) : Product  RndMeth=Zero
  Trigonometric Function{,1,6..8,11..12} (7 alike) : Trigonometry  Operator=cos
  Trigonometric Function{2..5,9..10,13} (7 alike) : Trigonometry
  Vector Concatenate : Concatenate  NumInputs=3  Mode=Multidimensional array
  Out1 : Outport
  wires:
  Trigonometric Function2/1 -> Product/1
  Trigonometric Function1/1 -> Product/2
  q/1 -> Trigonometric Function/1, Trigonometric Function2/1, Trigonometric Function3/1, Trigonometric Function5/1, Trigonometric Function6/1, Trigonometric Function8/1, Trigonometric Function12/1, Trigonometric Function13/1
  alpha/1 -> Trigonometric Function1/1, Trigonometric Function4/1, Trigonometric Function7/1, Trigonometric Function9/1, Trigonometric Function10/1, Trigonometric Function11/1
  Trigonometric Function3/1 -> Product1/1
  Trigonometric Function4/1 -> Product1/2
  Trigonometric Function6/1 -> Product2/1
  Trigonometric Function7/1 -> Product2/2
  Trigonometric Function8/1 -> Product3/1
  Trigonometric Function9/1 -> Product3/2
  Product3/1 -> Gain1/1
  Product/1 -> Gain/1
  Trigonometric Function/1 -> A_01/1
  Gain/1 -> A_01/2
  Product1/1 -> A_01/3
  Trigonometric Function5/1 -> A_01/4
  Product2/1 -> A_01/5
  Gain1/1 -> A_01/6
  Constant/1 -> A_01/7
  Trigonometric Function10/1 -> A_01/8
  Trigonometric Function11/1 -> A_01/9
  a/1 -> Product4/1, Product5/1
  Trigonometric Function12/1 -> Product4/2
  Trigonometric Function13/1 -> Product5/2
  Product4/1 -> Vector Concatenate/1
  Product5/1 -> Vector Concatenate/2
  Constant5/1 -> Vector Concatenate/3
  A_01/1 -> Matrix Concatenation/1
  Vector Concatenate/1 -> Matrix Concatenation/2
  Matrix Concatenation/1 -> Matrix Concatenation1/1
  Constant1/1 -> Matrix Concatenation1/2
  Matrix Concatenation1/1 -> Out1/1, Display/1

== system Simulatore_COMAU_UBHAND_IV_manipolazione_interna/Work Space Control/Inverse_kinematic/Inverse_kinematic_comau/first solution/k ==
  q1 : Inport
  P06 : Inport  Port=2
  Add1 : Sum  Inputs=-++
  Add2 : Sum  Inputs=+-
  Add3 : Sum  Inputs=+--
  Add4 : Sum  Inputs=+-
  Add5 : Sum  Inputs=+-
  Angle Conversion1 : aerolibconvert2/Angle Conversion  IU=rad  OU=deg
  Angle Conversion2 : aerolibconvert2/Angle Conversion  IU=rad  OU=deg
  Angle Conversion3 : aerolibconvert2/Angle Conversion  IU=rad  OU=deg
  Constant1 : Constant  Value=2
  Constant2 : Constant  Value=a_c(2)
  Constant3 : Constant  Value=dc4_1
  Constant4 : Constant  Value=450
  Constant6 : Constant  Value=3*pi/2
  Constant7 : Constant  Value=a_c(1)
  Constant8 : Constant  Value=teta_d
  Display{,1..9} (10 alike) : Display
  Divide2 : Product  Inputs=**
  Divide3 : Product  Inputs=**
  Divide4 : Product  Inputs=***
  Math Function{1..3,5..6} (5 alike) : Math  Operator=square  SaturateOnIntegerOverflow=on
  Math Function4 : Math  Operator=sqrt  SaturateOnIntegerOverflow=on
  P06(1) : dspmtrx3/Submatrix  RowSpan=One row  RowStartMode=Index  RowStartIndex=1  RowEndMode=Index  RowEndIndex=3  ColSpan=One column  ColStartMode=Index  ColStartIndex=1  ColEndMode=Offset from last  ColEndIndex=1
  P06(2) : dspmtrx3/Submatrix  RowSpan=One row  RowStartMode=Index  RowStartIndex=2  RowEndMode=Index  RowEndIndex=3  ColSpan=One column  ColStartMode=Index  ColStartIndex=1  ColEndMode=Offset from last  ColEndIndex=1
  P06(3) : dspmtrx3/Submatrix  RowSpan=One row  RowStartMode=Index  RowStartIndex=3  RowEndMode=Index  RowEndIndex=3  ColSpan=One column  ColStartMode=Index  ColStartIndex=1  ColEndMode=Offset from last  ColEndIndex=1
  Trigonometric Function : Trigonometry
  Trigonometric Function1 : Trigonometry  Operator=cos
  Trigonometric Function2 : Trigonometry  Operator=acos
  cos_teta : Product  Inputs=*/
  e_quadro : Sum  Inputs=+++
  teta1 : Gain  Gain=-1
  teta2 : Gain  Gain=-1
  q3 : Outport
  e_quadro1 : Outport  Port=2
  wires:
  Math Function3/1 -> e_quadro/3
  Math Function2/1 -> e_quadro/2
  Math Function1/1 -> e_quadro/1
  P06/1 -> P06(1)/1, P06(2)/1, P06(3)/1
  P06(1)/1 -> Add4/1
  q1/1 -> Trigonometric Function1/1, Trigonometric Function/1
  Constant7/1 -> Divide2/1, Divide3/1
  Trigonometric Function1/1 -> Divide2/2
  Divide2/1 -> Add4/2
  Trigonometric Function/1 -> teta1/1
  P06(2)/1 -> Add5/1
  Divide3/1 -> Add5/2
  Add5/1 -> Math Function2/1, Display1/1
  Add4/1 -> Math Function1/1, Display/1
  Constant2/1 -> Divide4/2, Math Function5/1
  Constant3/1 -> Divide4/3, Math Function6/1
  e_quadro/1 -> Add1/1, Math Function4/1, e_quadro1/1
  Add1/1 -> cos_teta/1
  Constant1/1 -> Divide4/1
  Divide4/1 -> cos_teta/2, Display8/1
  Constant8/1 -> Add3/2, Angle Conversion3/1
  Trigonometric Function2/1 -> Angle Conversion1/1, Add3/3, Angle Conversion2/1
  Add3/1 -> teta2/1
  Angle Conversion1/1 -> Display3/1
  Angle Conversion2/1 -> Display4/1
  Math Function4/1 -> Display5/1
  teta1/1 -> Divide3/2
  Constant6/1 -> Add3/1
  cos_teta/1 -> Trigonometric Function2/1, Display7/1
  Angle Conversion3/1 -> Display6/1
  P06(3)/1 -> Add2/1
  Constant4/1 -> Add2/2
  Add2/1 -> Math Function3/1, Display2/1
  Math Function5/1 -> Add1/2
  Math Function6/1 -> Add1/3, Display9/1
  teta2/1 -> q3/1
